simulink model "TosAeb"  (R2021a)
settings: Solver=FixedStepDiscrete  StartTime=0.0  StopTime=inf  FixedStep=0.01  RelTol=1e-3
undefined symbols (not defined in the file or in its folder): ACCModSts, AccSts1, AEB_ObjectMovingOrientation, AEB_ObjectMovingState, CN_BTCActive, CN_CurrentGearR, CN_CurrentGearR_DHT, CN_PTCActive, CN_VDCActive, CN_VSC_EspOff, CN_VSC_FCW_AEB_Error, CN_VSC_FCW_AEB_NoDispay, CN_VSC_FCW_AEB_PerfmDgdtn, CN_VSC_FCW_AEB_TempUnAvlbl, CN_VSC_VehStandstill, CNaccelAvoidMax, CNaccelAvoidMin, CNbrakePedalPress, CNcomfortStrLeftAccelMax, CNcomfortStrLeftAccelMin, CNcomfortStrRightAccelMax, CNcomfortStrRightAccelMin, CNDrvDoorOpen, CNDrvModeEco, CNDrvModeNorm, CNDrvModeOther, CNDrvModeSport, CNDrvSBRreminder, CNNull, CNobjInvalidID, CNPassDoorOpen, CNPassSBRreminder, CNPEBSAccelNegInf, CNPEBSAccelNull, CNPEBSTimeInf, CNPEBSTimeNull, CNSTOPMAX, CNSTOPNULL, CNsubjWidth, CNSwtOFF, CNSwtON, CNTTCMAX, CNTTCNULL, CNVehConfigAEBCycNone, CNVehConfigAEBNone, CNVehConfigAEBPedNone, CNVehConfigFCWNone, CNVehConfigJANone, CollisionSeverity, FALSE, FCWSnvty, JACycObjCalcInfoDefaultParams, JAPedObjCalcInfoDefaultParams, JAVehObjCalcInfoDefaultParams, latCycObjCalcInfoDefaultParams, latPedObjCalcInfoDefaultParams, longObjCalcInfoDefaultParams, ModSts, movingStatus, ObjectType ... (+1112)
libraries: simulink x3031, AEBSFunctionLib x50, Ta_Library x33, AEBSStateMachineLib x23, Mylibrary x12, simulink_extras x7, ObjSelect_lib x3, dspstat3 x2
(part 99 of 102)

== stateflow chart TosAeb/FcwAebJa_Step_sys/ALG_AEBSystem/ALG_AEBController/AEB_VehicleStateCalcApp/FuncErrorStateJudge/FCW_AEB_PedFctStsCalc ==
  note: 0x00:No display
      | 0x01:error
      | 0x02:FCW/AEB_V temporarily unavailable
      | 0x03:FCW/AEB_V Performance degradation
  (default) -> (junction 80)
  (junction 80) -> (junction 82) [order 1] : [FCW_AEB_ErrorState == TRUE]
  (junction 80) -> (junction 84) [order 2]
  (junction 82) -> (junction 5) : {
      | 	FCW_AEB_PedFctSts = ...
      | CN_VSC_FCW_AEB_Error;
      | }
  (junction 84) -> (junction 92) [order 1] : [EspFuncOffSts == CN_VSC_EspOff]
  (junction 84) -> (junction 90) [order 2]
  (junction 92) -> (junction 88) : {
      | 	FCW_AEB_PedFctSts = ...
      | CN_VSC_FCW_AEB_PerfmDgdtn;
      | }
  (junction 90) -> (junction 86) [order 1] : [TrailerSts == TRUE]
  (junction 90) -> (junction 98) [order 2] : {
      | 	FCW_AEB_PedFctSts = ...
      | CN_VSC_FCW_AEB_NoDispay;
      | }
  (junction 86) -> (junction 94) : {
      | 	FCW_AEB_PedFctSts = ...
      | CN_VSC_FCW_AEB_TempUnAvlbl;
      | }
  (junction 94) -> (junction 98)
  (junction 88) -> (junction 94)
  (junction 5) -> (junction 88)
  (junction 98) -> (junction 103)
  data: FCW_AEB_PedFctSts output uint8; FCW_AEB_ErrorState input; CN_VSC_FCW_AEB_TempUnAvlbl parameter; EspFuncOffSts input; TrailerSts input; TRUE parameter; CN_VSC_FCW_AEB_PerfmDgdtn parameter; CN_VSC_FCW_AEB_NoDispay parameter; CN_VSC_FCW_AEB_Error parameter; CN_VSC_EspOff parameter

== stateflow chart TosAeb/FcwAebJa_Step_sys/ALG_AEBSystem/ALG_AEBController/AEB_VehicleStateCalcApp/FuncErrorStateJudge/FCW_AEB_VehFctStsCalc ==
  note: 0x00:No display
      | 0x01:error
      | 0x02:FCW/AEB_V temporarily unavailable
      | 0x03:FCW/AEB_V Performance degradation
  (default) -> (junction 80)
  (junction 80) -> (junction 82) [order 1] : [FCW_AEB_ErrorState == TRUE]
  (junction 80) -> (junction 84) [order 2]
  (junction 82) -> (junction 5) : {
      | 	FCW_AEB_VehFctSts = ...
      | CN_VSC_FCW_AEB_Error;
      | }
  (junction 84) -> (junction 92) [order 1] : [EspFuncOffSts == CN_VSC_EspOff]
  (junction 84) -> (junction 90) [order 2]
  (junction 92) -> (junction 88) : {
      | 	FCW_AEB_VehFctSts = ...
      | CN_VSC_FCW_AEB_PerfmDgdtn;
      | }
  (junction 90) -> (junction 86) [order 1] : [TrailerSts == TRUE]
  (junction 90) -> (junction 98) [order 2] : {
      | 	FCW_AEB_VehFctSts = ...
      | CN_VSC_FCW_AEB_NoDispay;
      | }
  (junction 86) -> (junction 94) : {
      | 	FCW_AEB_VehFctSts = ...
      | CN_VSC_FCW_AEB_TempUnAvlbl;
      | }
  (junction 94) -> (junction 98)
  (junction 88) -> (junction 94)
  (junction 5) -> (junction 88)
  (junction 98) -> (junction 103)
  data: FCW_AEB_VehFctSts output uint8; FCW_AEB_ErrorState input; CN_VSC_FCW_AEB_TempUnAvlbl parameter; EspFuncOffSts input; TrailerSts input; TRUE parameter; CN_VSC_FCW_AEB_PerfmDgdtn parameter; CN_VSC_FCW_AEB_NoDispay parameter; CN_VSC_FCW_AEB_Error parameter; CN_VSC_EspOff parameter

== matlab function TosAeb/AEB_Objsel/TA_Processor/PreProcessor/ObjectPrecessor/TAObjInfoCalc/For Each Subsystem/Info_Check1/State_check/State_Classify2 ==
  data: ID_Change input; Fus_ObjState output; Fus_ObsType input; ObsID_u8 input; ObsMeasureStatus_u8 input; ObsVelXAbs_s input; ObsVelYAbs_s input; Spd_Stay input
function Fus_ObjState = fcn(ID_Change, Fus_ObsType, ObsID_u8, ObsMeasureStatus_u8, ObsVelXAbs_s, ObsVelYAbs_s, Spd_Stay)

%Fus_ObjState 目标运动状态
% 0：unknown 1:Moving 2:Stationary
% 3: Stopped 4:Moving_Slowly
ObjState = uint8(0);
%判断目标ID是否有效
if ID_Change == true || ObsID_u8 == uint8(0)
    ObjState = uint8(0);
else
    
    objSpd = sqrt(ObsVelXAbs_s*ObsVelXAbs_s + ObsVelYAbs_s*ObsVelYAbs_s);
     %对目标类型为车辆的运动状态进行校核
    if Fus_ObsType > uint8(0) && Fus_ObsType < uint8(9)
        if ObsMeasureStatus_u8 == uint8(1)
            if objSpd > single(2.5)
                ObjState = uint8(1);
            elseif objSpd <= single(0.5) && Spd_Stay <= single(0.5)
                ObjState = uint8(2);
            elseif objSpd <= single(0.5) && Spd_Stay > single(0.5)
                ObjState = uint8(3);
            elseif objSpd >= single(0.5) && objSpd <= single(2.5)
                ObjState = uint8(4);
            else
                ObjState = uint8(0);
            end
        elseif ObsMeasureStatus_u8 >=uint8(2) && ObsMeasureStatus_u8 <= uint8(5)
            ObjState = ObsMeasureStatus_u8-uint8(1);
        else
            ObjState = uint8(0);
        end
        %计算行人的运动状态
    else
        if objSpd > single(2.5) 
             ObjState = uint8(1);
        elseif objSpd <= single(0.5) && Spd_Stay <= single(0.5)
            ObjState = uint8(2);
        elseif objSpd <= single(0.5) && Spd_Stay > single(0.5)
            ObjState = uint8(3);
        elseif objSpd >= single(0.5) && objSpd <= single(2.5)
            ObjState = uint8(4);
        else
            ObjState = uint8(0);
        end
    end
end
Fus_ObjState = ObjState;
end

== stateflow chart TosAeb/AEB_Objsel/TA_Processor/PreProcessor/ObjectPrecessor/TAObjInfoCalc/For Each Subsystem/Info_Check1/State_check/Stop_Memory ==
  state init
    en:
    Spd_Stay = single(0);
  state Memory
    en:
    Spd_Stay = Spd;
  (default) -> init
  init -> Memory : [
      | ID ~= uint8(0)&&...
      | ID_Change == false
      | ]
  Memory -> init : [ID== uint8(0)]
  data: ID_Change input boolean; ID input uint8; Spd input single; Spd_Stay output single; timer local uint16

== matlab function TosAeb/AEB_Objsel/TA_Processor/PreProcessor/ObjectPrecessor/TAObjInfoCalc/For Each Subsystem/Info_Check1/Type_Precheck/Type_Classify1 ==
  data: ID_Change input; ObsID_u8 input; Fus_ObjType output; ObsMotionCategory_u8 input; ObsMotionStatus_u8 input; ObsClass_u8 input; ObsVhSubType_u8 input; ObsPedSubType_u8 input; ObsPedPos_u8 input; ObsLength_s input; ObsWidth_s input; ObsSpd_s input; P_TA_PrmForMotorSpdThred input
function Fus_ObjType = fcn(ID_Change,ObsID_u8,ObsMotionCategory_u8,ObsMotionStatus_u8,ObsClass_u8,ObsVhSubType_u8,ObsPedSubType_u8,ObsPedPos_u8,ObsLength_s,ObsWidth_s,ObsSpd_s,P_TA_PrmForMotorSpdThred)
%Fus_ObjType 传感器输出目标类型，对应参数如下：
% 0: unknown  1: Bus  2: Small_Medium_Car (中小型轿车) 3: Trucks (大卡车)  4: Motors (三轮车)
% 5: Special_vehicle (特殊车) 6: Tiny_car (微型车) 7: Lorry (小货车) 8: MiniVan (面包车)
% 9: Motor Cyclist(摩托车) 10：Cyclist（自行车）11：Pedestrian(Adult) 12:Pedestrian（child）
% 13: others(动物或不能划分清楚的)

%输出目标类型
%0x0: Unclear
%0x1: Bus
%0x2: Car
%0x3: Truck
%0x4: Motor Bike
%0x5: Bicycle
%0x6: Pedestrian
%0x7: Animals
%%
ObjType = uint8(0);
%判断目标的有效性，如果目标无效，目标类型为unknown
if ID_Change == true || ObsID_u8 == uint8(0)
    ObjType = uint8(0);
%当目标ID在1~200之间，即为车辆时，目标类型输出
elseif ObsID_u8 >= uint8(1)&& ObsID_u8 <= uint8(201)
    if ObsClass_u8 == uint8(1) && ObsMotionCategory_u8 ~= uint8(0) && ObsMotionStatus_u8 ~= uint8(0)
        if ObsVhSubType_u8 == uint8(1)  
            ObjType = uint8(1);
        elseif ObsVhSubType_u8 == uint8(2) || ObsVhSubType_u8 == uint8(8) || ObsVhSubType_u8 == uint8(6) || ObsVhSubType_u8 == uint8(4)
            ObjType = uint8(2);
        elseif ObsVhSubType_u8 == uint8(3) || ObsVhSubType_u8 == uint8(5) || ObsVhSubType_u8 == uint8(7)
            ObjType = uint8(3);
         else
            ObjType = uint8(0);
        end
    else
        ObjType = uint8(0);
    end
else
    if ObsClass_u8 == uint8(2) 
        if ObsPedSubType_u8 == uint8(0) || ObsPedPos_u8 == uint8(0)
            ObjType = uint8(0);
        else
            if ObsPedSubType_u8 == uint8(1) && ObsPedPos_u8 ~= uint8(0) && ObsPedPos_u8 ~= uint8(2)
                ObjType = uint8(6);
            elseif ObsPedSubType_u8 == uint8(1) && ObsPedPos_u8 ~= uint8(0) && ObsPedPos_u8 == uint8(2)
                if ObsSpd_s >= P_TA_PrmForMotorSpdThred
                    ObjType = uint8(4);
                else
                    ObjType = uint8(5);
                end
            elseif ObsPedSubType_u8 == uint8(2) && ObsPedPos_u8 ~= uint8(0) && ObsPedPos_u8 ~= uint8(2)
                ObjType = uint8(6);
            elseif ObsPedSubType_u8 == uint8(2) && ObsPedPos_u8 ~= uint8(0) && ObsPedPos_u8 == uint8(2)
               if ObsSpd_s >= P_TA_PrmForMotorSpdThred
                    ObjType = uint8(4);
                else
                    ObjType = uint8(5);
               end
            end
        end
    end
end
%由于未注明两轮车是属于行人还是车辆（目标ID处未给出明确划分），故在此单独处理
if ID_Change == false && ObsClass_u8 == uint8(3)
    if ObsLength_s ~= single(0) && ObsWidth_s ~= single(0) && ObsSpd_s >= P_TA_PrmForMotorSpdThred
         ObjType = uint8(4);
    elseif ObsLength_s ~= single(0) && ObsWidth_s ~= single(0) && ObsSpd_s < P_TA_PrmForMotorSpdThred
        ObjType = uint8(5);
    end
end   
Fus_ObjType = ObjType;
end

== stateflow chart TosAeb/FcwAebJa_Step_sys/ALG_AEBSystem/ALG_AEBController/AEB_JaObjCalcApp/JAPedTgtInfoCalc/JAPedObjCalcInfoCalc/JAPedObjInfoCalc/ObjCrossFlgCal/CollisionProbCalc/Subsystem/ObjLenthWidthCalc/ObjLengthWidthCalc ==
  (default) -> (junction 2)
  (junction 2) -> (junction 3) [order 1] : [ObjectTypeCameraConfirmed == true]
  (junction 2) -> (junction 7) [order 2] : {
      | 	ObjLengthCorr = 0.5;
      | 	ObjWidthCorr = 0.5;
      | }
  (junction 3) -> (junction 5) : {
      | 	ObjLengthCorr = ObjectLength;
      | 	ObjWidthCorr = ObjectWidth;
      | }
  (junction 5) -> (junction 7)
  (junction 7) -> (junction 10)
  (junction 10) -> (junction 12) [order 1] : [(abs(ObjectYawAngle) > 45 &&...
      | abs(ObjectYawAngle) < 135) ||...
      | objectMovingOrientation == AEB_ObjectMovingOrientation.Crossing]
  (junction 10) -> (junction 16) [order 2]
  (junction 12) -> (junction 14) : {
      | 	%ObjLengthCorr = ObjectWidth;
      | 	%ObjWidthCorr = ObjectLength;
      | ObjLengthCorr = ObjectLength;
      | ObjWidthCorr = ObjectWidth;
      | }
  (junction 14) -> (junction 16)
  (junction 16) -> (junction 19)
  data: ObjectYawAngle input single; objectMovingOrientation input; ObjectLength input single; ObjectTypeCameraConfirmed input; ObjectWidth input single; ObjLengthCorr output single; ObjWidthCorr output single

== stateflow chart TosAeb/FcwAebJa_Step_sys/ALG_AEBSystem/ALG_AEBController/AEB_JaObjCalcApp/JAPedTgtInfoCalc/JAPedObjCalcInfoCalc/JAPedObjInfoCalc/ObjCrossFlgCal/CollisionProbCalc/Subsystem/PreCrossFlgCalcFlow ==
  state preCrossFlg = VectorCrossAlgorithm(x1,y1,x2,y2,m1,n1,m2,n2) [function]
  (default) -> (junction 59) : {
      | 	preCrossFlg = false;
      | 	i = 0;
      | }
  (junction 59) -> (junction 58) [order 1] : [ i < 4]
  (junction 58) -> (junction 48) : {
      | 	p = (i+1)%%4;
      | 	j = 0;
      | }
  (junction 48) -> (junction 46) [order 1] : [j < 4]
  (junction 46) -> (junction 49) : {
      | 	q = (j+1)%%4;
      | 	preCrossFlg = VectorCrossAlgorithm(subjDxPre[i],subjDyPre[i],subjDxPre[p],subjDyPre[p],...
      | 	objDxPre[j],objDyPre[j],objDxPre[q],objDyPre[q]);
      | }
  (junction 60) -> (junction 59) : {
      | 	i++;
      | }
  (junction 59) -> (junction 61) [order 2]
  (junction 49) -> (junction 48) [order 2] : {
      | 	j++;
      | }
  (junction 48) -> (junction 60) [order 2]
  (junction 49) -> (junction 64) [order 1] : [preCrossFlg == true]
  (junction 64) -> (junction 61)
  (junction 61) -> (junction 67)
  data: x1 function_input single; y1 function_input single; x2 function_input single; y2 function_input single; m1 function_input single; n1 function_input single; m2 function_input single; n2 function_input single; preCrossFlg function_output; subjDxPre input single; subjDyPre input single; objDxPre input single; objDyPre input single; j local single; i local single; p local single; q local single; preCrossFlg output boolean

== stateflow chart TosAeb/FcwAebJa_Step_sys/ALG_AEBSystem/ALG_AEBController/AEB_JaObjCalcApp/JAPedTgtInfoCalc/JAPedObjCalcInfoCalc/JAPedObjInfoCalc/ObjCrossFlgCal/CollisionProbCalcFlow ==
  (default) -> (junction 11)
  (junction 11) -> (junction 2) : {tPredictTime = 0;}
  (junction 2) -> (junction 6) [order 1] : [tPredictTime <= P_JaPedCA_PreCollisionPredTimeMax]
  (junction 6) -> (junction 8) : {
      | 	tPredictTimeOut = tPredictTime;
      | 	CollisionProbFunc;
      | }
  (junction 8) -> (junction 2) [order 2] : {
      | 	tPredictTime += P_JaPedCA_PreCollisionStepTime;
      | }
  (junction 2) -> (junction 13) [order 2] : {
      |   TTC = single(256);
      | }
  (junction 8) -> (junction 22) [order 1] : [preCollisionProb == true]
  (junction 22) -> (junction 13) : {
      |   TTC = tPredictTimeOut;
      | }
  (junction 13) -> (junction 25)
  data: TTC output single; P_JaPedCA_PreCollisionStepTime input single; P_JaPedCA_PreCollisionPredTimeMax input single; tPredictTimeOut output single; tPredictTime local single; preCollisionProb input
  events: CollisionProbFunc output FUNCTION_CALL_EVENT

== stateflow chart TosAeb/FcwAebJa_Step_sys/ALG_AEBSystem/ALG_AEBController/AEB_JaObjCalcApp/JAPedTgtInfoCalc/TargetSelectCalc/tgtSelectFlow/tgtObjFlow ==
  state TargetSelectFunc [function]
    note: change dy to dx
    (default) -> (junction 63) : {
        | 	tempObjPreSelectCond = ObjPreSelectCondFufilled(index);
        | 	tempFlg = JaPedObjCalcInfo(index).AEBJAPedObjColliInfo.ObjColliFlg;
        | }
    (junction 63) -> (junction 75) [order 1] : [tempObjPreSelectCond== true &&...
        | tempFlg == true &&...
        | abs(SteerWheelAngle_Deg) > P_JaPedCA_SelectStrWheelAngleAbsThres &&...
        | subjVel <= P_JaPedCA_SelectSubjVelThres]
    (junction 63) -> (junction 69) [order 2]
    (junction 75) -> (junction 311)
    (junction 311) -> (junction 312) [order 1] : [ObjPreSelectCondFufilled(index) == false]
    (junction 311) -> (junction 318) [order 2]
    (junction 312) -> (junction 315)
    (junction 318) -> (junction 321) [order 1] : [ObjPreSelectCondFufilled(tgtIndex) == false ||...
        | JaPedObjCalcInfo(index).AEBJAPedObjColliInfo.ObjColliFlg == false]
    (junction 318) -> (junction 128) [order 2] : {
        | 	tgtIsFind = true;
        | 	temp1dx = abs(JaPedObjCalcInfo(index).AEBJAPedObj.dx);
        | 	temp2dx = abs(JaPedObjCalcInfo(tgtIndex).AEBJAPedObj.dx);
        | 	temp1t = JaPedObjCalcInfo(index).AEBJAPedObjColliInfo.tPredictTime;
        | 	temp2t = JaPedObjCalcInfo(tgtIndex).AEBJAPedObjColliInfo.tPredictTime;
        | }
    (junction 321) -> (junction 323) : {
        | 	tgtIndex = index;
        | }
    (junction 128) -> (junction 131) [order 1] : [temp1t < temp2t]
    (junction 128) -> (junction 292) [order 2] : {
        | 	temp = abs(temp1t - temp2t);
        | }
    (junction 131) -> (junction 289) : {tgtIndex = index;}
    (junction 292) -> (junction 294) [order 1] : [temp < 1e-6&&...
        |  temp1dx < temp2dx]
    (junction 292) -> (junction 101) [order 2]
    (junction 294) -> (junction 296) : {tgtIndex = index;}
    (junction 101) -> (junction 69)
    (junction 296) -> (junction 101)
    (junction 289) -> (junction 296)
    (junction 323) -> (junction 289)
    (junction 315) -> (junction 323)
    (junction 69) -> (junction 81)
  (default) -> (junction 111) : {
      | 	tgtIsFind = false;
      | }
  (junction 111) -> (junction 201) [order 1] : [TargetIDK1 ~=  CNobjInvalidID]
  (junction 111) -> (junction 286) [order 2]
  (junction 201) -> (junction 236)
  (junction 236) -> (junction 238) [order 1] : [JAPedPrefillModSts == ModSts.ActiveState ||...
      | JAPedAEBfullModSts == ModSts.ActiveState ]
  (junction 236) -> (junction 284) [order 2]
  (junction 238) -> (junction 282) : {
      | 	tgtIndex = tgtIndexK1;
      | 	tgtIsFind = true;
      | }
  (junction 284) -> (junction 286)
  (junction 286) -> (junction 112) : {
      | 	tgtIndex = uint8(1);
      | 	index = uint8(1);
      | }
  (junction 112) -> (junction 110) [order 1] : [index <= 32]
  (junction 113) -> (junction 112) : {index = index + 1;}
  (junction 112) -> (junction 114) [order 2]
  (junction 110) -> (junction 113) : {
      | 	TargetSelectFunc();
      | }
  (junction 282) -> (junction 114)
  (junction 114) -> (junction 220)
  (junction 220) -> (junction 222) [order 1] : [tgtIsFind == true]
  (junction 220) -> (junction 223) [order 2] : {
      | 	JaPedTgtObjInfo = JAPedObjCalcInfoDefaultParams;
      | }
  (junction 222) -> (junction 221) : {
      | 	JaPedTgtObjInfo = JaPedObjCalcInfo(tgtIndex);
      | }
  (junction 221) -> (junction 223)
  (junction 223) -> (junction 224)
  data: JaPedObjCalcInfo input Bus: BusAEBJAPedObjCalcInfo size=32; index local uint8; JaPedTgtObjInfo output Bus: BusAEBJAPedObjCalcInfo; temp2t local single; temp1t local single; ObjPreSelectCondFufilled input boolean size=32; subjVel input single; SteerWheelAngle_Deg input single; JAPedAEBfullModSts input Enum: ModSts; JAPedPrefillModSts input Enum: ModSts; TargetIDK1 input uint16; tgtIndex output uint8; tgtIndexK1 input uint8; tgtIsFind local; JAPedObjCalcInfoDefaultParams parameter Bus: BusAEBJAPedObjCalcInfo; CNobjInvalidID parameter single; temp1dx local single; temp2dx local single; tempFlg local; tempObjPreSelectCond local boolean; P_JaPedCA_SelectStrWheelAngleAbsThres input single; P_JaPedCA_SelectSubjVelThres input single

== stateflow chart TosAeb/FcwAebJa_Step_sys/ALG_AEBSystem/ALG_AEBController/AEB_JaObjCalcApp/JAVehTgtInfoCalc/AEBJAVehObjCalc/JAVehObjInfoCalc/ObjCrossFlgCal/CollisionProbCalc/Subsystem/ObjLenthWidthCalc/ObjLengthWidthCalc ==
  (default) -> (junction 2)
  (junction 2) -> (junction 3) [order 1] : [FusionType == true]
  (junction 2) -> (junction 7) [order 2] : {
      | 	ObjLengthCorr = P_JaVehCA_PrecollisionVehLengthThres;
      | 	ObjWidthCorr = P_JaVehCA_PrecollisionVehWidthThres;
      | }
  (junction 3) -> (junction 49)
  (junction 49) -> (junction 32) [order 1] : [ObjectLength<P_JaVehCA_PrecollisionVehLengthThres]
  (junction 49) -> (junction 38) [order 2] : {ObjLengthCorr = P_JaVehCA_PrecollisionVehLengthThres;}
  (junction 32) -> (junction 51)
  (junction 51) -> (junction 53) [order 1] : [ObjectLength<0.5]
  (junction 38) -> (junction 40) [order 1] : [ObjectWidth<P_JaVehCA_PrecollisionVehWidthThres]
  (junction 51) -> (junction 34) [order 2] : {ObjLengthCorr = ObjectLength;}
  (junction 53) -> (junction 55) : {ObjLengthCorr =0.5; }
  (junction 38) -> (junction 5) [order 2] : {ObjWidthCorr = P_JaVehCA_PrecollisionVehWidthThres;}
  (junction 40) -> (junction 58)
  (junction 58) -> (junction 60) [order 1] : [ObjectWidth<0.5]
  (junction 58) -> (junction 44) [order 2] : {ObjWidthCorr = ObjectWidth;}
  (junction 60) -> (junction 62) : {ObjWidthCorr = 0.5;}
  (junction 5) -> (junction 7)
  (junction 44) -> (junction 5)
  (junction 62) -> (junction 44)
  (junction 34) -> (junction 62)
  (junction 55) -> (junction 34)
  (junction 7) -> (junction 10)
  (junction 10) -> (junction 12) [order 1] : [(abs(ObjectYawAngle) > 45 &&...
      | abs(ObjectYawAngle) < 135) ||...
      | objectMovingOrientation == AEB_ObjectMovingOrientation.Crossing]
  (junction 10) -> (junction 16) [order 2]
  (junction 12) -> (junction 14) : {
      |   ObjLengthCorr = ObjectWidth;
      |   ObjWidthCorr = ObjectLength;
      | }
  (junction 14) -> (junction 16)
  (junction 16) -> (junction 19)
  data: ObjectYawAngle input single; objectMovingOrientation input; ObjectLength input single; FusionType input; ObjectWidth input single; ObjLengthCorr output single; ObjWidthCorr output single; P_JaVehCA_PrecollisionVehLengthThres input; P_JaVehCA_PrecollisionVehWidthThres input

== stateflow chart TosAeb/FcwAebJa_Step_sys/ALG_AEBSystem/ALG_AEBController/AEB_JaObjCalcApp/JAVehTgtInfoCalc/AEBJAVehObjCalc/JAVehObjInfoCalc/ObjCrossFlgCal/CollisionProbCalc/Subsystem/PreCrossFlgCalcFlow ==
  state preCrossFlg = VectorCrossAlgorithm(x1,y1,x2,y2,m1,n1,m2,n2) [function]
  (default) -> (junction 59) : {
      | 	preCrossFlg = false;
      | 	i = 0;
      | }
  (junction 59) -> (junction 58) [order 1] : [ i < 4]
  (junction 58) -> (junction 48) : {
      | 	p = (i+1)%%4;
      | 	j = 0;
      | }
  (junction 48) -> (junction 46) [order 1] : [j < 4]
  (junction 46) -> (junction 49) : {
      | 	q = (j+1)%%4;
      | 	preCrossFlg = VectorCrossAlgorithm(subjDxPre[i],subjDyPre[i],subjDxPre[p],subjDyPre[p],...
      | 	objDxPre[j],objDyPre[j],objDxPre[q],objDyPre[q]);
      | }
  (junction 60) -> (junction 59) : {
      | 	i++;
      | }
  (junction 59) -> (junction 61) [order 2]
  (junction 49) -> (junction 48) [order 2] : {
      | 	j++;
      | }
  (junction 48) -> (junction 60) [order 2]
  (junction 49) -> (junction 64) [order 1] : [preCrossFlg == true]
  (junction 64) -> (junction 61)
  (junction 61) -> (junction 67)
  data: x1 function_input single; y1 function_input single; x2 function_input single; y2 function_input single; m1 function_input single; n1 function_input single; m2 function_input single; n2 function_input single; preCrossFlg function_output; subjDxPre input single; subjDyPre input single; objDxPre input single; objDyPre input single; j local single; i local single; p local single; q local single; preCrossFlg output boolean

== stateflow chart TosAeb/FcwAebJa_Step_sys/ALG_AEBSystem/ALG_AEBController/AEB_JaObjCalcApp/JAVehTgtInfoCalc/AEBJAVehObjCalc/JAVehObjInfoCalc/ObjCrossFlgCal/CollisionProbCalcFlow ==
  (default) -> (junction 11)
  (junction 11) -> (junction 2) : {tPredictTime = 0;}
  (junction 2) -> (junction 6) [order 1] : [tPredictTime <= P_JaVehCA_PreCollisionPredTimeMax]
  (junction 8) -> (junction 2) [order 2] : {
      | 	tPredictTime = tPredictTime+P_JaVehCA_PreCollisionStepTime;
      | }
  (junction 2) -> (junction 13) [order 2]
  (junction 6) -> (junction 8) : {
      | 	tPredictTimeOut = tPredictTime;
      | 	CollisionProbFunc;
      | }
  (junction 8) -> (junction 22) [order 1] : [preCollisionProb == true]
  (junction 22) -> (junction 13)
  (junction 13) -> (junction 25) : {
      |  TTC = tPredictTimeOut;
      | }
  data: TTC output single; P_JaVehCA_PreCollisionStepTime input single; P_JaVehCA_PreCollisionPredTimeMax input single; tPredictTimeOut output single; tPredictTime local single; preCollisionProb input
  events: CollisionProbFunc output FUNCTION_CALL_EVENT

== stateflow chart TosAeb/FcwAebJa_Step_sys/ALG_AEBSystem/ALG_AEBController/AEB_FuncCondCalcApp/LongFunctionCondCalc/vehEBASuppAborCondCalc/vehEBASuppCondCalc/vehEBASituationRelActiveCondCalc/brakeReleventSuppCond/brakeReleventSuppCond_IBooster/brkTriggerCondCalc1 ==
  state MaxDecel = brkAccelThesLookup(subjVel) [function]
  (default) -> (junction 2)
  (junction 2) -> (junction 3) [order 1] : [objMovingState == AEB_ObjectMovingState.Still]
  (junction 2) -> (junction 28) [order 2] : {
      | 	%brkAccelThes = temp_brkAccelThes1;
      | brkAccelThes =brkAccelThesLookup(subjVel);
      | }
  (junction 3) -> (junction 14)
  (junction 14) -> (junction 16) [order 1] : [predictiveAccelAvoid < objStandAccelAvoidPredictThes]
  (junction 14) -> (junction 24) [order 2] : {
      | 	brkAccelThes =...
      |  	objStandBrkAccelThesLow;
      | }
  (junction 16) -> (junction 11) : {
      | 	brkAccelThes = ...
      | 	objStandBrkAccelThesHigh;
      | }
  (junction 24) -> (junction 28)
  (junction 11) -> (junction 24)
  (junction 28) -> (junction 31)
  (junction 31) -> (junction 48) [order 1] : [
      | 	IB_BrkPedalAppld == true && ...
      | 	subjLongAccelFromSensor < brkAccelThes && ...
      | 	subjLongAccel < subjLongAccelThes
      | ]
  (junction 31) -> (junction 52) [order 2] : {
      | 	brkTriggerCondFulfilled = false;
      | }
  (junction 48) -> (junction 50) : {
      | 	brkTriggerCondFulfilled = true;
      | }
  (junction 50) -> (junction 52)
  (junction 52) -> (junction 55)
  data: subjVel function_input single; MaxDecel function_output; predictiveAccelAvoid input; objMovingState input; subjVel input; brkTriggerCondFulfilled output boolean; subjLongAccelFromSensor input; subjLongAccel input; IB_BrkPedalAppld input; objStandAccelAvoidPredictThes input; objStandBrkAccelThesHigh input; objStandBrkAccelThesLow input; subjLongAccelThes input; brkAccelThes local single

== stateflow chart TosAeb/FcwAebJa_Step_sys/ALG_AEBSystem/ALG_AEBController/AEB_JaObjCalcApp/JAVehTgtInfoCalc/TargetFinalSelect/tgtSelect/tgtObjFlow ==
  state TargetSelectFunc [function]
    note: dy replaced with dx
    (default) -> (junction 248) : {
        |     tempObjPreSelectCond = ObjPreSelectCondFufilled(index);
        |     tempFlg = JAVehObjCalcInfo(index).AEBJAVehObjColliInfo.ObjColliFlg;
        | }
    (junction 248) -> (junction 249) [order 1] : [tempObjPreSelectCond == true &&...
        | tempFlg == true &&...
        | abs(SteerWheelAngle_Deg) >=P_JaVehCA_SelectStrWheelAngleAbsThres &&...
        | subjVel <= P_JaVehCA_SelectSubjVelThres]
    (junction 248) -> (junction 252) [order 2]
    (junction 249) -> (junction 274) : {
        |   tgtIsFind = true;
        | }
    (junction 274) -> (junction 276) [order 1] : [ObjPreSelectCondFufilled(tgtIndex) == false||...
        | JAVehObjCalcInfo(tgtIndex).AEBJAVehObjColliInfo.ObjColliFlg == false]
    (junction 274) -> (junction 247) [order 2] : {
        | 	temp1 = abs(JAVehObjCalcInfo(index).AEBJAVehObj.dx);
        | 	temp2 = abs(JAVehObjCalcInfo(tgtIndex).AEBJAVehObj.dx);
        | 	temp1t = JAVehObjCalcInfo(index).AEBJAVehObjColliInfo.tPredictTime;
        | 	temp2t = JAVehObjCalcInfo(tgtIndex).AEBJAVehObjColliInfo.tPredictTime;
        | }
    (junction 276) -> (junction 278) : {
        | 	tgtIndex = index;
        | }
    (junction 247) -> (junction 251) [order 1] : [temp1 < temp2]
    (junction 251) -> (junction 255) : {
        | 	tgtIndex = index;
        | }
    (junction 247) -> (junction 253) [order 2] : {
        |    temp = abs(temp1t - temp2t);
        | }
    (junction 253) -> (junction 250) [order 1] : [ temp <1e-6&&...
        |   temp1t <= temp2t]
    (junction 253) -> (junction 256) [order 2]
    (junction 250) -> (junction 254) : {
        | 	tgtIndex = index;
        | }
    (junction 256) -> (junction 252)
    (junction 254) -> (junction 256)
    (junction 255) -> (junction 254)
    (junction 278) -> (junction 255)
    (junction 252) -> (junction 257)
  (default) -> (junction 111) : {
      | 	tgtIsFind = false;
      | }
  (junction 111) -> (junction 197) [order 1] : [TargetIDK1 ~=  CNobjInvalidID]
  (junction 111) -> (junction 215) [order 2]
  (junction 197) -> (junction 199)
  (junction 199) -> (junction 215) [order 2]
  (junction 199) -> (junction 201) [order 1] : [JAVehPrefillModSts == ModSts.ActiveState ||...
      | JAVehAEBfullModSts == ModSts.ActiveState ]
  (junction 215) -> (junction 112) : {
      | 	tgtIndex = uint8(1);
      | 	index = uint8(1);
      | }
  (junction 201) -> (junction 203) : {
      | 	tgtIndex = tgtIndexK1;
      | 	tgtIsFind = true;
      | }
  (junction 112) -> (junction 110) [order 1] : [index <= 32]
  (junction 113) -> (junction 112) : {index = index + 1;}
  (junction 112) -> (junction 212) [order 2]
  (junction 110) -> (junction 113) : {
      | 	TargetSelectFunc();
      | }
  (junction 203) -> (junction 212)
  (junction 212) -> (junction 114)
  (junction 114) -> (junction 205) [order 1] : [tgtIsFind == true]
  (junction 114) -> (junction 182) [order 2] : {
      | 	JAVehTgtObjInfo = JAVehObjCalcInfoDefaultParams;
      | }
  (junction 205) -> (junction 207) : {
      | 	JAVehTgtObjInfo = JAVehObjCalcInfo(tgtIndex);
      | }
  (junction 207) -> (junction 182)
  (junction 182) -> (junction 210)
  data: JAVehObjCalcInfo input Bus: BusAEBJAVehObjCalcInfo size=32; index local uint8; JAVehTgtObjInfo output Bus: BusAEBJAVehObjCalcInfo; temp2 local single; temp1 local single; ObjPreSelectCondFufilled input boolean size=32; subjVel input; SteerWheelAngle_Deg input; JAVehAEBfullModSts input Enum: ModSts; JAVehPrefillModSts input Enum: ModSts; P_JaVehCA_SelectSubjVelThres input; P_JaVehCA_SelectStrWheelAngleAbsThres input; TargetIDK1 input; tgtIndexK1 input uint8; JAVehObjCalcInfoDefaultParams parameter Bus: BusAEBJAVehObjCalcInfo; CNobjInvalidID parameter uint8; tgtIndex output uint8; tempFlg local; tempObjPreSelectCond local; temp1t local; temp2t local; tgtIsFind local boolean

== matlab function TosAeb/AEB_Objsel/TA_Processor/PreProcessor/VehiclePreProcessor/CalculateCurvature/StaticBicycleModelC1/IntermediateCalculation/SafeDivide/SafeDivide ==
  data: Numerator input; Quotient output; Denominator input; DataTypeMin parameter; DataTypeMax parameter
function Quotient = fcn(Numerator, Denominator, DataTypeMin, DataTypeMax)
    %#codegen
    
    % Test for division by zero and overflow
    if abs(Denominator) > abs(Numerator)*(1/DataTypeMax)
        % Normal divide
        Quotient=Numerator/Denominator;
    else
        % Select output depending on the numerator
        if Numerator>0
            % x/0
            Quotient=DataTypeMax;
        elseif Numerator<0
            % -x/0
            Quotient=DataTypeMin;
        else
            % 0/0
            Quotient=cast(0,class(Numerator));
        end
    end
end

== matlab function TosAeb/AEB_Objsel/TA_Processor/PreProcessor/VehiclePreProcessor/PathEstimn_Calculate/For Iterator PahEstimn/ComputeVehicleStateAtT1/MATLAB Function ==
  data: A input; VLgt output; Speed input; HeadingAngle input; Curvature input; VLat output; ALgt output; ALat output
function [VLgt,VLat,ALgt,ALat] = fcn(A,Speed,HeadingAngle,Curvature)
%#codegen

% Perpendicular acceleration from speed and curvature.
AccelerationPerpendicular = single(Curvature*Speed*Speed);

% Convert acceleration to cartesian coordinate system 
ALgt = single( -AccelerationPerpendicular*sin(HeadingAngle) + A*cos(HeadingAngle));
ALat = single(AccelerationPerpendicular*cos(HeadingAngle) + A*sin(HeadingAngle));

% Convert speed to cartesian coordinate system
VLgt = single(cos(HeadingAngle)*Speed);
VLat = single(sin(HeadingAngle)*Speed);

== matlab function TosAeb/AEB_Objsel/TA_Processor/PreProcessor/VehiclePreProcessor/PathEstimn_Calculate/For Iterator PahEstimn/InitialControlPointsCalculation/MATLAB Function ==
  data: PositionLongitudinal input; P3Longitudinal output; PositionLateral input; V input; A input; HeadingAngle input; Curvature input; CurvatureRate input; StopsWithinSegment input; LongitudinalStoppingDistance input; LateralStoppingDistance input; P3Lateral output
function [P3Longitudinal,P3Lateral] = fcn(PositionLongitudinal, PositionLateral, V, A, HeadingAngle, ...
    Curvature, CurvatureRate, StopsWithinSegment, LongitudinalStoppingDistance, LateralStoppingDistance)
%#codegen

% Calculate the position using constant curvature c0 and c0+c1, respectively.
% Then make a weighted average.
% The correct position can not be found analytically, thus the weight factor
% is determined using simulations.

DetectableCurvature = single(1e-5);
if abs(Curvature) > DetectableCurvature && abs(Curvature + CurvatureRate) > DetectableCurvature
    
    % Distance at time 1 s, which equals the arc length.
    distance = A/2 + V;                     % a0*t^2/2 + v0*t, for t = 1.
    
    % Curvature radius.
    r1 = 1/Curvature;          
    r2 = 1/(Curvature + CurvatureRate);
    angleOfArc1 = distance/r1;
    angleOfArc2 = distance/r2;

    % Position in coordinate system rotated with heading h0. 
    xrot1 = r1*sin(angleOfArc1);            % Position if radius = 1/c0.
    yrot1 = -r1*cos(angleOfArc1) + r1;
    xrot2 = r2*sin(angleOfArc2);            % Position if radius = 1/(c0+c1).
    yrot2 = -r2*cos(angleOfArc2) + r2;
    
    % Rotate back to the normal coordinate system.
    x1 = xrot1*cos(HeadingAngle) - yrot1*sin(HeadingAngle);
    y1 = xrot1*sin(HeadingAngle) + yrot1*cos(HeadingAngle);
    x2 = xrot2*cos(HeadingAngle) - yrot2*sin(HeadingAngle);
    y2 = xrot2*sin(HeadingAngle) + yrot2*cos(HeadingAngle);

    % Longitudinal and lateral position at time 1 s.
    P3Longitudinal = PositionLongitudinal + 0.7*x1 + 0.3*x2;
    P3Lateral = PositionLateral + 0.7*y1 + 0.3*y2;
    
else
    P3Longitudinal = PositionLongitudinal + (A/2 + V)*(cos(HeadingAngle));
    P3Lateral = PositionLateral + (A/2 + V)*(sin(HeadingAngle));   
end

% If vehicle stops, use the stopping distance for calculating the predicted position.
if StopsWithinSegment
    P3Longitudinal = PositionLongitudinal + LongitudinalStoppingDistance;
    P3Lateral = PositionLateral + LateralStoppingDistance;
end

== stateflow chart TosAeb/FcwAebJa_Step_sys/ALG_AEBSystem/ALG_AEBController/AEB_LatObjInfoCalcApp/LatCycObjCalc/LatCycCollisonAssessInfoCalc/LatCycCollisonAssessInfoCalc/LatCollsionSeverityJudge/LatCollsionSeverityJudgeFlow ==
  note: 变更1和2的条件
  (default) -> (junction 88)
  (junction 88) -> (junction 89) [order 1] : [ latCollisionSeverityK1== CollisionSeverity.Low]
  (junction 89) -> (junction 90) : {
      | 	collisionSeverityLowThres = ...
      | P_LatCycCA_Lvl1SeverityThresCurrentLvl0;
      | 	collisionSeverityMediumThres = ...
      | P_LatCycCA_Lvl2SeverityThresCurrentLvl0;
      | 	collisionSeverityHignThres = ...
      | P_LatCycCA_Lvl3SeverityThresCurrentLvl0;
      | }
  (junction 88) -> (junction 92) [order 2]
  (junction 92) -> (junction 93) [order 1] : [ latCollisionSeverityK1== CollisionSeverity.Low]
  (junction 92) -> (junction 96) [order 2]
  (junction 93) -> (junction 94) : {
      | 	collisionSeverityLowThres = ...
      | P_LatCycCA_Lvl1SeverityThresCurrentLvl1;
      | 	collisionSeverityMediumThres = ...
      | P_LatCycCA_Lvl2SeverityThresCurrentLvl1;
      | 	collisionSeverityHignThres = ...
      | P_LatCycCA_Lvl3SeverityThresCurrentLvl1;
      | }
  (junction 96) -> (junction 97) [order 1] : [ latCollisionSeverityK1== CollisionSeverity.Medium]
  (junction 96) -> (junction 100) [order 2] : {
      | 	collisionSeverityLowThres = ...
      | P_LatCycCA_Lvl1SeverityThresCurrentLvl3;
      | 	collisionSeverityMediumThres = ...
      | P_LatCycCA_Lvl2SeverityThresCurrentLvl3;
      | 	collisionSeverityHignThres = ...
      | P_LatCycCA_Lvl3SeverityThresCurrentLvl3;
      | }
  (junction 97) -> (junction 98) : {
      | 	collisionSeverityLowThres = ...
      | P_LatCycCA_Lvl1SeverityThresCurrentLvl2;
      | 	collisionSeverityMediumThres = ...
      | P_LatCycCA_Lvl2SeverityThresCurrentLvl2;
      | 	collisionSeverityHignThres = ...
      | P_LatCycCA_Lvl3SeverityThresCurrentLvl2;
      | }
  (junction 90) -> (junction 94)
  (junction 94) -> (junction 98)
  (junction 98) -> (junction 100)
  (junction 100) -> (junction 106)
  (junction 106) -> (junction 108) [order 1] : [ObjectID == CNobjInvalidID]
  (junction 108) -> (junction 144) : {
      | 	tempLatCollsionSeverity = 0;
      | }
  (junction 106) -> (junction 124) [order 2]
  (junction 124) -> (junction 120) [order 1] : [ cycColliisonRiskProb > collisionSeverityHignThres]
  (junction 124) -> (junction 187) [order 2]
  (junction 120) -> (junction 158) : {
      | 	tempLatCollsionSeverity = 3;
      | }
  (junction 187) -> (junction 113) [order 1] : [ cycColliisonRiskProb > collisionSeverityMediumThres]
  (junction 187) -> (junction 196) [order 2]
  (junction 113) -> (junction 112) : {
      | 	tempLatCollsionSeverity = 2;
      | }
  (junction 196) -> (junction 136) [order 1] : [ cycColliisonRiskProb > collisionSeverityLowThres]
  (junction 196) -> (junction 119) [order 2] : {
      | 	tempLatCollsionSeverity = 0;
      | }
  (junction 136) -> (junction 174) : {
      | 	tempLatCollsionSeverity = 1;
      | }
  (junction 158) -> (junction 112)
  (junction 144) -> (junction 158)
  (junction 112) -> (junction 174)
  (junction 174) -> (junction 119)
  (junction 119) -> (junction 132) : {
      | 	latCollsionSeverity = tempLatCollsionSeverity;
      | }
  data: ObjectID input; cycColliisonRiskProb input single; latCollsionSeverity output Enum: CollisionSeverity; collisionSeverityLowThres local single; collisionSeverityMediumThres local single; collisionSeverityHignThres local single; tempLatCollsionSeverity local Enum: CollisionSeverity; CNobjInvalidID parameter; P_LatCycCA_Lvl1SeverityThresCurrentLvl0 input; P_LatCycCA_Lvl2SeverityThresCurrentLvl0 input; P_LatCycCA_Lvl3SeverityThresCurrentLvl0 input; P_LatCycCA_Lvl1SeverityThresCurrentLvl1 input; P_LatCycCA_Lvl2SeverityThresCurrentLvl1 input; P_LatCycCA_Lvl3SeverityThresCurrentLvl1 input; P_LatCycCA_Lvl1SeverityThresCurrentLvl2 input; P_LatCycCA_Lvl2SeverityThresCurrentLvl2 input; P_LatCycCA_Lvl3SeverityThresCurrentLvl2 input; P_LatCycCA_Lvl1SeverityThresCurrentLvl3 input; P_LatCycCA_Lvl2SeverityThresCurrentLvl3 input; P_LatCycCA_Lvl3SeverityThresCurrentLvl3 input; latCollisionSeverityK1 input Enum: CollisionSeverity

== stateflow chart TosAeb/FcwAebJa_Step_sys/ALG_AEBSystem/ALG_AEBController/AEB_LatObjInfoCalcApp/LatCycObjCalc/LatCycObjCalc/latCycObjInfoCalc/ObjColiisionRiskProbCalc/CollisionRiskProbCalc/CollisionRiskProbCalc/dxOverlapFlow ==
  (default) -> (junction 32) : {
      | 	dxOverlapLimit = dxReginObjRUCorr - dxReginObjLLCorr;
      | 	dxOverlapLimit = max(dxOverlapLimit,0.1);
      | }
  (junction 32) -> (junction 34) [order 1] : [dxReginObjLLCorr > dxReginEgoRUCorr || ...
      | dxReginObjRUCorr < dxReginEgoLLCorr]
  (junction 32) -> (junction 2) [order 2]
  (junction 34) -> (junction 36) : {
      | 	dxOverlap = 0;
      | }
  (junction 2) -> (junction 3) [order 1] : [dxReginEgoRUCorr - dxReginEgoLLCorr < dxOverlapLimit && ...
      | dxReginEgoLLCorr >= dxReginObjLLCorr &&...
      | dxReginEgoRUCorr < dxReginObjRUCorr]
  (junction 2) -> (junction 7) [order 2]
  (junction 3) -> (junction 5) : {
      | 	dxOverlap = dxReginEgoRUCorr - dxReginEgoLLCorr;
      | }
  (junction 7) -> (junction 9) [order 1] : [dxReginEgoRUCorr - dxReginObjLLCorr < dxOverlapLimit &&...
      | dxReginEgoRUCorr - dxReginObjLLCorr > 0 && ...
      | dxReginEgoLLCorr < dxReginObjLLCorr]
  (junction 7) -> (junction 13) [order 2]
  (junction 9) -> (junction 11) : {
      | 	dxOverlap = dxReginEgoRUCorr - dxReginObjLLCorr;
      | }
  (junction 13) -> (junction 15) [order 1] : [dxReginObjRUCorr - dxReginEgoLLCorr< dxOverlapLimit && ...
      | dxReginObjRUCorr - dxReginEgoLLCorr> 0 && ...
      | dxReginEgoRUCorr > dxReginObjRUCorr]
  (junction 13) -> (junction 19) [order 2] : {
      | 	dxOverlap= dxOverlapLimit;
      | }
  (junction 15) -> (junction 17) : {
      | 	dxOverlap = dxReginObjRUCorr - dxReginEgoLLCorr;
      | }
  (junction 17) -> (junction 19)
  (junction 11) -> (junction 17)
  (junction 5) -> (junction 11)
  (junction 36) -> (junction 5)
  (junction 19) -> (junction 22)
  data: dxReginEgoRUCorr input; dxReginEgoLLCorr input; dxReginObjRUCorr input; dxReginObjLLCorr input; dxOverlap output single; dxOverlapLimit output single

== stateflow chart TosAeb/FcwAebJa_Step_sys/ALG_AEBSystem/ALG_AEBController/AEB_LatObjInfoCalcApp/LatCycObjCalc/LatCycObjCalc/latCycObjInfoCalc/ObjColiisionRiskProbCalc/CollisionRiskProbCalc/CollisionRiskProbCalc/dyOverlapFlow ==
  (default) -> (junction 32) : {
      | 	dyOverlapLimit = dyReginObjLLCorr- dyReginObjRUCorr;
      | 	dyOverlapLimit = max(dyOverlapLimit,0.1);
      | }
  (junction 32) -> (junction 34) [order 1] : [dyReginObjLLCorr < dyReginEgoRUCorr || ...
      | dyReginObjRUCorr > dyReginEgoLLCorr]
  (junction 32) -> (junction 2) [order 2]
  (junction 34) -> (junction 36) : {
      | 	dyOverlap = 0;
      | }
  (junction 2) -> (junction 3) [order 1] : [dyReginEgoLLCorr - dyReginEgoRUCorr < dyOverlapLimit && ...
      | dyReginEgoLLCorr <= dyReginObjLLCorr &&...
      | dyReginEgoRUCorr >= dyReginObjRUCorr]
  (junction 2) -> (junction 7) [order 2]
  (junction 3) -> (junction 5) : {
      | 	dyOverlap = dyReginEgoLLCorr - dyReginEgoRUCorr;
      | }
  (junction 7) -> (junction 9) [order 1] : [dyReginObjLLCorr - dyReginEgoRUCorr < dyOverlapLimit &&...
      | dyReginObjLLCorr - dyReginEgoRUCorr >0 && ...
      | dyReginEgoLLCorr > dyReginObjLLCorr]
  (junction 7) -> (junction 13) [order 2]
  (junction 9) -> (junction 11) : {
      | 	dyOverlap = dyReginObjLLCorr - dyReginEgoRUCorr;
      | }
  (junction 13) -> (junction 15) [order 1] : [dyReginEgoLLCorr - dyReginObjRUCorr < dyOverlapLimit && ...
      | dyReginEgoLLCorr - dyReginObjRUCorr > 0 && ...
      | dyReginEgoRUCorr < dyReginObjRUCorr]
  (junction 13) -> (junction 19) [order 2] : {
      | 	dyOverlap= dyOverlapLimit;
      | }
  (junction 15) -> (junction 17) : {
      | 	dyOverlap = dyReginEgoLLCorr - dyReginObjRUCorr;
      | }
  (junction 17) -> (junction 19)
  (junction 11) -> (junction 17)
  (junction 5) -> (junction 11)
  (junction 36) -> (junction 5)
  (junction 19) -> (junction 22)
  data: dyReginEgoRUCorr input; dyReginEgoLLCorr input; dyReginObjRUCorr input; dyReginObjLLCorr input; dyOverlap output single; dyOverlapLimit output single

== matlab function TosAeb/FcwAebJa_Step_sys/ALG_AEBSystem/ALG_AEBController/AEB_LatObjInfoCalcApp/LatCycObjCalc/LatCycObjCalc/latCycObjInfoCalc/ObjColiisionRiskProbCalc/CollisionRiskProbCalc/MATLAB Function ==
  data: dxReginEgoRUCorr input; dyReginEgoRUCorr input; dyReginEgoLLCorr input; dxReginEgoLLCorr input; dxReginObjRUCorr input; dyReginObjRUCorr input; dxReginObjLLCorr input; dyReginObjLLCorr input; dx input; dy input; collisionRiskProb input; dyCollisionRiskProb input; dxCollisionRiskProb input
function Overlappatch(dxReginEgoRUCorr, dyReginEgoRUCorr, dyReginEgoLLCorr, dxReginEgoLLCorr,...
    dxReginObjRUCorr, dyReginObjRUCorr, dxReginObjLLCorr, dyReginObjLLCorr,...
    dx, dy, collisionRiskProb, dyCollisionRiskProb, dxCollisionRiskProb)
coder.extrinsic('patch')
coder.extrinsic('num2str')
coder.extrinsic('plot')

persistent ii
if (isempty(ii))
    ii = 1;
end
dxReginEgoLUCorr = dxReginEgoRUCorr;
dyReginEgoLUCorr = dyReginEgoLLCorr;
dxReginEgoRLCorr = dxReginEgoLLCorr;
dyReginEgoRLCorr = dyReginEgoRUCorr;

dxReginObjLUCorr = dxReginObjRUCorr;
dyReginObjLUCorr = dyReginObjLLCorr;
dxReginObjRLCorr = dxReginObjLLCorr;
dyReginObjRLCorr = dyReginObjRUCorr;

xEgo = [dxReginEgoRUCorr,dxReginEgoLUCorr,dxReginEgoLLCorr,dxReginEgoRLCorr];
yEgo = [dyReginEgoRUCorr,dyReginEgoLUCorr,dyReginEgoLLCorr,dyReginEgoRLCorr];
xObj = [dxReginObjRUCorr,dxReginObjLUCorr,dxReginObjLLCorr,dxReginObjRLCorr];
yObj = [dyReginObjRUCorr,dyReginObjLUCorr,dyReginObjLLCorr,dyReginObjRLCorr];
cla
% cla reset

patch(xEgo,yEgo,'-o',...
    'LineWidth',1','FaceColor','red','EdgeColor','red','FaceAlpha',.2)
% hold on
patch(xObj,yObj,'-o',...
    'LineWidth',1','FaceColor','blue','EdgeColor','blue','FaceAlpha',.4)

txtEgoRU = ['RU(',num2str(dxReginEgoRUCorr),',',num2str(dyReginEgoRUCorr),')'];
text(dxReginEgoRUCorr,dyReginEgoRUCorr,txtEgoRU,'Color','red','FontSize',10)
txtEgoLL = ['LL(',num2str(xEgo(3)),',',num2str(yEgo(3)),')'];
text(xEgo(3),yEgo(3),txtEgoLL,'Color','red','FontSize',10)

txtObjRU = ['RU(',num2str(xObj(1)),',',num2str(yObj(1)),')'];
text(xObj(1),yObj(1),txtObjRU,'Color','blue','FontSize',10)
txtObjLL = ['LL(',num2str(xObj(3)),',',num2str(yObj(3)),')'];
text(xObj(3),yObj(3),txtObjLL,'Color','blue','FontSize',10)

% text(sum(xObj)/4,sum(yObj)/4,['dxOverlap:', num2str(dxCollisionRiskProb)])
% text(sum(xObj)/4+0.1,sum(yObj)/4,['dyOverlap:',num2str(dyCollisionRiskProb)])


% a = ii;
% b = ii;
% 
% x(1,a) = dx;
% y(1,b) = dy;
% hold on;
% % plot(x(1:a),y(1:b),'k--o','LineWidth',2)
% plot(dx,dy,'k--o','LineWidth',2)
% 
ii = ii + 1;
if (ii > 20)
    ii = 1;
    close all;
end
end
% pause(0.5);

== stateflow chart TosAeb/FcwAebJa_Step_sys/ALG_AEBSystem/ALG_AEBController/AEB_LatObjInfoCalcApp/LatCycObjCalc/LatCycObjCalc/latCycObjInfoCalc/ObjColiisionRiskProbCalc/CollisionRiskProbCalc/ObjReginCorrCalc/ObjLengthWidthCalc/ObjLengthWidthCalc ==
  (default) -> (junction 10) : {
      | 	ObjLengthCorr = 1.8;
      | 	ObjWidthCorr = 0.5;
      | }
  (junction 10) -> (junction 12) [order 1] : [(abs(ObjectYawAngle) > 45 &&...
      | abs(ObjectYawAngle) < 135)||...
      | ObjectMovingOrientation == AEB_ObjectMovingOrientation.Crossing]
  (junction 10) -> (junction 16) [order 2]
  (junction 12) -> (junction 14) : {
      | 	ObjLengthCorr = ObjectWidth;
      | 	ObjWidthCorr = ObjectLength;
      | }
  (junction 14) -> (junction 16)
  (junction 16) -> (junction 19)
  data: ObjectWidth input; ObjectYawAngle input; ObjectMovingOrientation input; ObjectLength input; ObjLengthCorr output single; ObjWidthCorr output single

== stateflow chart TosAeb/FcwAebJa_Step_sys/ALG_AEBSystem/ALG_AEBController/AEB_LatObjInfoCalcApp/LatCycObjCalc/LatCycObjCalc/latCycObjInfoCalc/ObjColiisionRiskProbCalc/CollisionRiskProbCalc/ObjReginCorrCalc/ObjLengthWidthCalc/ObjLengthWidthCalc1 ==
  (default) -> (junction 32)
  (junction 32) -> (junction 34) [order 1] : [ObjectTypeCameraConfirmed == true]
  (junction 32) -> (junction 30) [order 2] : {
      | 	ObjectLength = P_LatCycCA_CollisionRiskProbLengthThres;
      | 	ObjectWidth = P_LatCycCA_CollisionRiskProbWidthThres;
      | }
  (junction 34) -> (junction 36) : {
      | 	ObjectLength = ObjectLengthIn;
      | 	ObjectWidth = ObjectWidthIn;
      | }
  (junction 36) -> (junction 30)
  (junction 30) -> (junction 10)
  (junction 10) -> (junction 12) [order 1] : [(abs(ObjectYawAngle) > 45 &&...
      | abs(ObjectYawAngle) < 135)||...
      | ObjectMovingOrientation == AEB_ObjectMovingOrientation.Crossing]
  (junction 10) -> (junction 16) [order 2]
  (junction 12) -> (junction 14) : {
      |       temp = ObjectLength;
      | 	ObjectLength = ObjectWidth;
      | 	ObjectWidth = temp;
      | }
  (junction 14) -> (junction 16)
  (junction 16) -> (junction 19) : {
      |    ObjectLength = min(ObjectLength,2.5);
      |    ObjectWidth = min(ObjectWidth,2.5);
      | }
  data: ObjectTypeCameraConfirmed input boolean; ObjectLengthIn input; ObjectWidthIn input; ObjectYawAngle input; ObjectMovingOrientation input; ObjectLength output single; ObjectWidth output single; P_LatCycCA_CollisionRiskProbLengthThres input; P_LatCycCA_CollisionRiskProbWidthThres input; temp local single

== stateflow chart TosAeb/FcwAebJa_Step_sys/ALG_AEBSystem/ALG_AEBController/AEB_LatObjInfoCalcApp/LatCycObjCalc/LatCycObjCalc/latCycObjInfoCalc/ObjColiisionRiskProbCalc/CollisionRiskProbCalc/ObjReginCorrCalc/ObjLengthWidthCalc1/ObjLengthWidthCalc ==
  (default) -> (junction 10) : {
      | 	ObjLengthCorr = P_LatPedCA_CollisionRiskProbLengthThres;
      | 	ObjWidthCorr = P_LatPedCA_CollisionRiskProbWidthThres;
      | }
  (junction 10) -> (junction 12) [order 1] : [(abs(ObjectYawAngle) > 45 &&...
      | abs(ObjectYawAngle) < 135)||...
      | ObjectMovingOrientation == AEB_ObjectMovingOrientation.Crossing]
  (junction 10) -> (junction 16) [order 2]
  (junction 12) -> (junction 14) : {
      | 	ObjLengthCorr = ObjectWidth;
      | 	ObjWidthCorr = ObjectLength;
      | }
  (junction 14) -> (junction 16)
  (junction 16) -> (junction 19)
  data: ObjectWidth input; ObjectYawAngle input; ObjectMovingOrientation input; ObjectLength input; ObjLengthCorr output single; ObjWidthCorr output single; P_LatPedCA_CollisionRiskProbLengthThres input; P_LatPedCA_CollisionRiskProbWidthThres input

== stateflow chart TosAeb/FcwAebJa_Step_sys/ALG_AEBSystem/ALG_AEBController/AEB_LatObjInfoCalcApp/LatCycObjCalc/LatCycObjCalc/latCycObjInfoCalc/ObjColiisionRiskProbCalc/CollisionRiskProbCalc/ObjReginCorrCalc/ObjLengthWidthCalc1/ObjLengthWidthCalc1 ==
  (default) -> (junction 32)
  (junction 32) -> (junction 34) [order 1] : [ObjectTypeCameraConfirmed == true]
  (junction 32) -> (junction 30) [order 2] : {
      | 	ObjectLength = P_LatPedCA_CollisionRiskProbLengthThres;
      | 	ObjectWidth = P_LatPedCA_CollisionRiskProbWidthThres;
      | }
  (junction 34) -> (junction 36) : {
      | 	ObjectLength = ObjectLengthIn;
      | 	ObjectWidth = ObjectWidthIn;
      | }
  (junction 36) -> (junction 30)
  (junction 30) -> (junction 10)
  (junction 10) -> (junction 12) [order 1] : [(abs(ObjectYawAngle) > 45 &&...
      | abs(ObjectYawAngle) < 135)||...
      | ObjectMovingOrientation == AEB_ObjectMovingOrientation.Crossing]
  (junction 10) -> (junction 16) [order 2]
  (junction 12) -> (junction 14) : {
      |       temp = ObjectLength;
      | 	ObjectLength = ObjectWidth;
      | 	ObjectWidth = temp;
      | }
  (junction 14) -> (junction 16)
  (junction 16) -> (junction 19) : {
      |    ObjectLength = min(ObjectLength,1.5);
      |    ObjectWidth = min(ObjectWidth,1.5);
      | }
  data: ObjectTypeCameraConfirmed input boolean; ObjectLengthIn input; ObjectWidthIn input; ObjectYawAngle input; ObjectMovingOrientation input; ObjectLength output single; ObjectWidth output single; P_LatPedCA_CollisionRiskProbLengthThres input; P_LatPedCA_CollisionRiskProbWidthThres input; temp local single
